simulink model "ofdm_txrx_supermimo"  (Simulink 7.1)
settings: Solver=VariableStepDiscrete  SolverMode=SingleTasking  StartTime=0.0  StopTime=simOnly_simLength  RelTol=1e-3
PostLoadFcn:
  | ofdm_rx_supermimo_init
InitFcn:
  | ofdm_rx_supermimo_init
StartFcn:
  | ofdm_rx_supermimo_init
values from neighbouring files (symbols this model uses):
  Action0_Config = 0* 31*2^18 + 0*2^24 + PHY_AUTORESPONSE_REQ_GOODHDR + PHY_AUTORESPONSE_REQ_GOODPKT + PHY_AUTORESPONSE_REQ_FLAGA    [ofdm_tx_supermimo_init.m]
  Action1_Config = 0* 62*2^18 + PHY_AUTORESPONSE_REQ_GOODHDR + PHY_AUTORESPONSE_REQ_GOODPKT    [ofdm_tx_supermimo_init.m]
  Action2_Config = 0    [ofdm_tx_supermimo_init.m]
  Action3_Config = 0    [ofdm_tx_supermimo_init.m]
  Action4_Config = 0    [ofdm_tx_supermimo_init.m]
  Action5_Config = 0    [ofdm_tx_supermimo_init.m]
  ActionID_AFTransmit = 31    [ofdm_tx_supermimo_init.m]
  ActionID_Disabled = 0    [ofdm_tx_supermimo_init.m]
  ActionID_SetFlagA = 62    [ofdm_tx_supermimo_init.m]
  ActionID_SetFlagB = 61    [ofdm_tx_supermimo_init.m]
  BER_RAM_init_size = 2048    [ofdm_tx_supermimo_init.m]
  CPLength = 16    [ofdm_tx_supermimo_init.m]
  longCorr_coef_i = [-4*real(LTS) 0]    [ofdm_rx_supermimo_init.m]
  longCorr_coef_q = [4*imag(LTS) 0]    [ofdm_rx_supermimo_init.m]
  Match0_Config = 0    [ofdm_tx_supermimo_init.m]
  Match1_Config = 0    [ofdm_tx_supermimo_init.m]
  Match2_Config = 0    [ofdm_tx_supermimo_init.m]
  Match3_Config = 0    [ofdm_tx_supermimo_init.m]
  Match4_Config = 0    [ofdm_tx_supermimo_init.m]
  Match5_Config = 0    [ofdm_tx_supermimo_init.m]
  Match6_Config = 0    [ofdm_tx_supermimo_init.m]
  Match7_Config = 0    [ofdm_tx_supermimo_init.m]
  max_CP_length = 16    [ofdm_tx_supermimo_init.m]
  max_num_baseRateSymbols = 31    [ofdm_tx_supermimo_init.m]
  max_num_subcarriers = 64    [ofdm_tx_supermimo_init.m]
  max_num_trainingSymbols = 15    [ofdm_tx_supermimo_init.m]
  max_numBytes = 16384    [ofdm_tx_supermimo_init.m]
  max_OFDM_symbols = 511    [ofdm_tx_supermimo_init.m]
  modConstellation_qam16 = .75*[1 3 -1 -3]./3    [ofdm_tx_supermimo_init.m]
  modConstellation_qam64 = 0.875*[3 1 5 7 -3 -1 -5 -7]./7    [ofdm_tx_supermimo_init.m]
  modConstellation_qpsk = [1 -1]./sqrt(2)    [ofdm_tx_supermimo_init.m]
  numBaseRateSymbols = ceil(numHeaderBytes / numBytes_BaseRateOFDMSymbol)    [ofdm_tx_supermimo_init.m]
  numSubcarriers = 64    [ofdm_tx_supermimo_init.m]
  numTrainingSymbols = 2    [ofdm_tx_supermimo_init.m]
  pilot_indicies = 7 + ( (64-21) * 2^8) + ( (64-7) * 2^16) + (21 * 2^24)    (= 356068103)    [ofdm_tx_supermimo_init.m]
  pilotValues = hex2dec('A57D') + (2^16 * hex2dec('5A82'))    [ofdm_tx_supermimo_init.m]
  pkt_crossCorr_thresh = 0.7    [ofdm_rx_supermimo_init.m]
  pkt_energy_thresh = 0    [ofdm_rx_supermimo_init.m]
  pktByteNums = numHeaderBytes +  				byteIndex_numPayloadBytes(1)*2^8 +  				byteIndex_numPayloadBytes(2)*2^16 +  				byteIndex_simpleDynModMasks * 2^24    [ofdm_rx_supermimo_init.m]
  PktDet_Delay = 58+32 +      Symbol_Timing_Offset*2^7 +      0*2^16 +      (117* 2^24)    (= 1962935642)    [ofdm_rx_supermimo_init.m]
  pktTiming_count_payloadStart = pktTiming_count_lsStart + pktTiming_ls_num*pktTiming_ls_length    [ofdm_rx_supermimo_init.m]
  preamble = preamble_scale*[shortsyms_10 longsyms_2]    [ofdm_tx_supermimo_init.m]
  preamble_ROM = preamble_I_ROM + preamble_Q_ROM*2^16    [ofdm_tx_supermimo_init.m]
  RAM_init_size = max_numBytes    (= 16384)    [ofdm_tx_supermimo_init.m]
  reg_AF_Tx_Blanking = (320+80) + ( (320+80+79)*2^16)    (= 31392144)    [ofdm_rx_supermimo_init.m]
  reg_AF_Tx_Scaling = 2944    [ofdm_rx_supermimo_init.m]
  reg_Interrupt_PktBuf_Control = reg_InterruptControl +  	1 * 2^16 +  	0 * 2^24    (= 65656)    [ofdm_rx_supermimo_init.m]
  reg_PilotCalcParams = (4 * 2^16) + (minPilotChanMag)    (= 262184.96)    [ofdm_rx_supermimo_init.m]
  regRx_chanEst_minMag = ((0.5)*2^15) + ((0.5 * 2^15)*2^16)    (= 1073758208)    [ofdm_rx_supermimo_init.m]
  regRx_coarseCalcCorrection = round((1.3e-3 - 1.2982e-3)*2^32)    [ofdm_rx_supermimo_init.m]
  regRx_pilotCalcCorrection = round(1.00115 * 2^31)    [ofdm_rx_supermimo_init.m]
  regRx_preCFOoptions = 0 * 2^0 +  	1 * 2^1 +  	0    (= 2)    [ofdm_rx_supermimo_init.m]
  rx_controlBits = 1 * 2  		 + 1 * 4  		 + 0 * 8  		 + 1 * 16 * rx_SISO_Mode  		 + 1 * 32  		 + 1 * 64  		 + 1 * 128  		 + 1 * 256  		 + 1 * 512  		 + 1 * 1024  		 + 1 * 4096  		 + 0 * 16384  		 + 0 * 32768  		 + 1 * 65536  		 + 0 * 131072  		 + 0 * 262144  		 + 1 * 524288  		 + 1 * 1048576 * tx_Alamouti_Mode  		 + 0 * 2097152           + 1 * 33554432  		 + 0 * 4194304  		 + 0 * 8388608  		 + 0 * 16777216  		 + 1 *  ...(+49 chars)    [ofdm_rx_supermimo_init.m]
  Rx_PktDet_LongCorr_Params = LongCorrCounterLoad + 0*2^8 + 0*2^16 + 255*2^24    (= 4278190325)    [ofdm_rx_supermimo_init.m]
  Rx_PktDet_LongCorrThresholds = 8000    [ofdm_rx_supermimo_init.m]
  rx_postEq_scaling = round(rxScaling*2^11) * (1 + 2^16)    [ofdm_rx_supermimo_init.m]
  RxDataScrambling_Seq = [40 198 78 63 82 173 102 245 48 111 172 115 147 230 216 93 72 65 62 2 205 242 122 90 128 83 105 97 73 10 5 252]    [ofdm_tx_supermimo_init.m]
  RxReg_FixedPktLen = 0    [ofdm_rx_supermimo_init.m]
  shortSymbol_time = shortSymbol_time(1:16).'    [ofdm_tx_supermimo_init.m]
  simOnly_numSamples = length(preamble)+( (numSubcarriers+CPLength)*(numTrainingSymbols + numBaseRateSymbols + numFullRateSymbols/2) )    [ofdm_tx_supermimo_init.m]
  train = [train train]    [ofdm_tx_supermimo_init.m]
  tx_controlBits = (antB_preambleShift * 2^4) + (2^1 * tx_Alamouti_Mode) +  	(2^0 * tx_SISO_Mode) + 4*0 + 8*1 + 0*256 + 512*0 + 1024*0 +  	0*hex2dec('1000') + 0*hex2dec('2000') + 0*hex2dec('4000') +  	1*hex2dec('8000') + 1*hex2dec('10000') + hex2dec('20000') +  	16*hex2dec('100000')    [ofdm_tx_supermimo_init.m]
  tx_delays = (50*2^0) + (0*2^8) + (30*2^12)    (= 122930)    [ofdm_tx_supermimo_init.m]
  tx_scaling = (3072) + (12288 * 2^16)    (= 805309440)    [ofdm_tx_supermimo_init.m]
  TxDataScrambling_Seq = [40 198 78 63 82 173 102 245 48 111 172 115 147 230 216 93 72 65 62 2 205 242 122 90 128 83 105 97 73 10 5 252]    [ofdm_tx_supermimo_init.m]
  txfilt_firpm = 0.95*txfilt_firpm./(max(txfilt_firpm))    [ofdm_tx_supermimo_init.m]
  txfilt_intfilt = [0 intfilt(4,4,.8)]    [ofdm_tx_supermimo_init.m]
  txReg_symbolCounts = (2^8 *numBaseRateSymbols ) + numTrainingSymbols    [ofdm_tx_supermimo_init.m]
  TxRx_FFTScaling = (3 * 2^0) +  	(2 * 2^2) +  	(1 * 2^4) +  	(1 * 2^6) +  	(1 * 2^8) +  	(0 * 2^10)    (= 347)    [ofdm_tx_supermimo_init.m]
undefined symbols (not defined in the file or in its folder): AntA, AntB, BaseRate, Down, gcbh, numBuffs, numBytes, Up, xlBlockLoadCallback, xlGetSimulinkPeriod, xlipgetpartsetting
libraries: xbsIndex_r4 x6035, simulink x4, dspsigops x4, commchan3 x2, dspsrcs4 x2, commrflib2 x2
(part 21 of 61)

== system ofdm_txrx_supermimo/OFDM Rx MIMO/FFT & Chan Est/FFT/FreqSelective "Fading" ==
  I : Inport
  Q : Inport  Port=2
  Ind : Inport  Port=3
  "FreqSelective \"Fading\"" : Subsystem
  Simulation Multiplexer : xbsIndex_r4/Simulation Multiplexer  sim_sel=1  hw_sel=2  block_type=simmux
      settings: infoedit="Distinguish input subsystems as \"simulation only\" and \"generation only\".  The input specified For Simulation will be used during Simulink simulation.  The input specified For Generation will be used during code generation.  This block will typically be used on the output of a HW Co-Simulation, Black Box, or ModelSim subsystem.<P><P>Hardware Notes: This block costs nothing."  block_version=10.1.3
  Simulation Multiplexer1 : xbsIndex_r4/Simulation Multiplexer  sim_sel=1  hw_sel=2  block_type=simmux
      settings: infoedit="Distinguish input subsystems as \"simulation only\" and \"generation only\".  The input specified For Simulation will be used during Simulink simulation.  The input specified For Generation will be used during code generation.  This block will typically be used on the output of a HW Co-Simulation, Black Box, or ModelSim subsystem.<P><P>Hardware Notes: This block costs nothing."  block_version=10.1.3
  " I" : Outport  OutDataType=sfix(16)  OutScaling=2^0
  " Q" : Outport  Port=2  OutDataType=sfix(16)  OutScaling=2^0
  wires:
  "FreqSelective \"Fading\""/1(" I") -> Simulation Multiplexer/1
  "FreqSelective \"Fading\""/2(" Q") -> Simulation Multiplexer1/1
  I/1 -> "FreqSelective \"Fading\""/1(I), Simulation Multiplexer/2
  Q/1 -> "FreqSelective \"Fading\""/2(Q), Simulation Multiplexer1/2
  Ind/1 -> "FreqSelective \"Fading\""/3(Ind)
  Simulation Multiplexer/1 -> " I"/1
  Simulation Multiplexer1/1 -> " Q"/1

== system ofdm_txrx_supermimo/OFDM Rx MIMO/FFT & Chan Est/Input Buffers/Antenna A RAM ==
  I : Inport  OutDataType=sfix(16)  OutScaling=2^0
  Q : Inport  Port=2  OutDataType=sfix(16)  OutScaling=2^0
  We : Inport  Port=3  OutDataType=sfix(16)  OutScaling=2^0
  Wr Addr : Inport  Port=4  OutDataType=sfix(16)  OutScaling=2^0
  Rd Addr : Inport  Port=5  OutDataType=sfix(16)  OutScaling=2^0
  Constant8 : xbsIndex_r4/Constant  arith_type=Boolean  const=0  n_bits=1  bin_pt=0  explicit_period=off  period=1  equ=P=C  opselect=C  inp2=PCIN>>17  opr=+  inp1=P  carry=CIN  dbl_ovrd=off  block_type=constant
      settings: block_version=VER_STRING_GOES_HERE
  Dual Port RAM : xbsIndex_r4/Dual Port RAM  depth=2*numSubcarriers  initVector=[0.01:0.01:0.80 ones(1,48)]  distributed_mem=Block RAM  init_a=0  init_b=0  rst_a=off  rst_b=off  en_a=off  en_b=off  latency=1  write_mode_A=Read After Write  write_mode_B=Read After Write  dbl_ovrd=off  optimize=Area  use_rpm=off  block_type=dpram
      settings: xl_area=[0 0 1 0 0 0 0]  block_version=VER_STRING_GOES_HERE
  Dual Port RAM1 : xbsIndex_r4/Dual Port RAM  depth=2*numSubcarriers  initVector=[0.80:-0.01:0.01 ones(1,48)]  distributed_mem=Block RAM  init_a=0  init_b=0  rst_a=off  rst_b=off  en_a=off  en_b=off  latency=1  write_mode_A=Read After Write  write_mode_B=Read After Write  dbl_ovrd=off  optimize=Area  use_rpm=off  block_type=dpram
      settings: xl_area=[0 0 1 0 0 0 0]  block_version=VER_STRING_GOES_HERE
  " I" : Outport  OutDataType=sfix(16)  OutScaling=2^0
  " Q" : Outport  Port=2  OutDataType=sfix(16)  OutScaling=2^0
  wires:
  Wr Addr/1 -> Dual Port RAM1/1, Dual Port RAM/1
  Constant8/1 -> Dual Port RAM/6, Dual Port RAM1/6
  Rd Addr/1 -> Dual Port RAM1/4, Dual Port RAM/4
  Dual Port RAM1/2 -> " Q"/1
  Dual Port RAM/2 -> " I"/1
  I/1 -> Dual Port RAM/2, Dual Port RAM/5, Dual Port RAM1/5
  Q/1 -> Dual Port RAM1/2
  We/1 -> Dual Port RAM1/3, Dual Port RAM/3

== system ofdm_txrx_supermimo/OFDM Rx MIMO/FFT & Chan Est/Input Buffers/Antenna B RAM ==
  I : Inport  OutDataType=sfix(16)  OutScaling=2^0
  Q : Inport  Port=2  OutDataType=sfix(16)  OutScaling=2^0
  We : Inport  Port=3  OutDataType=sfix(16)  OutScaling=2^0
  Wr Addr : Inport  Port=4  OutDataType=sfix(16)  OutScaling=2^0
  Rd Addr : Inport  Port=5  OutDataType=sfix(16)  OutScaling=2^0
  Constant8 : xbsIndex_r4/Constant  arith_type=Boolean  const=0  n_bits=1  bin_pt=0  explicit_period=off  period=1  equ=P=C  opselect=C  inp2=PCIN>>17  opr=+  inp1=P  carry=CIN  dbl_ovrd=off  block_type=constant
      settings: block_version=VER_STRING_GOES_HERE
  Dual Port RAM : xbsIndex_r4/Dual Port RAM  depth=2*numSubcarriers  initVector=[0.01:0.01:0.80 ones(1,48)]  distributed_mem=Block RAM  init_a=0  init_b=0  rst_a=off  rst_b=off  en_a=off  en_b=off  latency=1  write_mode_A=Read After Write  write_mode_B=Read After Write  dbl_ovrd=off  optimize=Area  use_rpm=off  block_type=dpram
      settings: xl_area=[0 0 1 0 0 0 0]  block_version=VER_STRING_GOES_HERE
  Dual Port RAM1 : xbsIndex_r4/Dual Port RAM  depth=2*numSubcarriers  initVector=[0.80:-0.01:0.01 ones(1,48)]  distributed_mem=Block RAM  init_a=0  init_b=0  rst_a=off  rst_b=off  en_a=off  en_b=off  latency=1  write_mode_A=Read After Write  write_mode_B=Read After Write  dbl_ovrd=off  optimize=Area  use_rpm=off  block_type=dpram
      settings: xl_area=[0 0 1 0 0 0 0]  block_version=VER_STRING_GOES_HERE
  " I" : Outport  OutDataType=sfix(16)  OutScaling=2^0
  " Q" : Outport  Port=2  OutDataType=sfix(16)  OutScaling=2^0
  wires:
  We/1 -> Dual Port RAM/3, Dual Port RAM1/3
  Q/1 -> Dual Port RAM1/2
  I/1 -> Dual Port RAM/2, Dual Port RAM/5, Dual Port RAM1/5
  Dual Port RAM/2 -> " I"/1
  Dual Port RAM1/2 -> " Q"/1
  Rd Addr/1 -> Dual Port RAM/4, Dual Port RAM1/4
  Constant8/1 -> Dual Port RAM1/6, Dual Port RAM/6
  Wr Addr/1 -> Dual Port RAM/1, Dual Port RAM1/1

== system ofdm_txrx_supermimo/OFDM Rx MIMO/FFT & Chan Est/Input Buffers/We Control ==
  note: In Alamouti mode, alternate symbols
      | are written to alternate memory buffers.
      | In MIMO mode, symbols are written to buffers
      | simultaneously (from each antenna).
  We : Inport
  WrSlot : Inport  Port=2
  Down Sample : xbsIndex_r4/Down Sample  sample_ratio=2  sample_phase="Last Value of Frame  (most efficient)"  en=off  latency=1  dbl_ovrd=off  block_type=dsamp
      settings: block_version=VER_STRING_GOES_HERE
  Inverter1 : xbsIndex_r4/Inverter  en=off  latency=0  dbl_ovrd=off  block_type=inv
      settings: block_version=VER_STRING_GOES_HERE
  Inverter2 : xbsIndex_r4/Inverter  en=off  latency=0  dbl_ovrd=off  block_type=inv
      settings: block_version=VER_STRING_GOES_HERE
  Inverter3 : xbsIndex_r4/Inverter  en=off  latency=0  dbl_ovrd=off  block_type=inv
      settings: block_version=VER_STRING_GOES_HERE
  Logical1 : xbsIndex_r4/Logical  logical_function=AND  inputs=3  en=off  latency=0  precision=Full  arith_type=Unsigned  n_bits=16  bin_pt=0  align_bp=on  dbl_ovrd=off  block_type=logical
      settings: block_version=8.2.02
  Logical3 : xbsIndex_r4/Logical  logical_function=AND  inputs=3  en=off  latency=0  precision=Full  arith_type=Unsigned  n_bits=16  bin_pt=0  align_bp=on  dbl_ovrd=off  block_type=logical
      settings: block_version=8.2.02
  Logical4 : xbsIndex_r4/Logical  logical_function=OR  inputs=2  en=off  latency=0  precision=Full  arith_type=Unsigned  n_bits=16  bin_pt=0  align_bp=on  dbl_ovrd=off  block_type=logical
      settings: block_version=8.2.02
  Logical5 : xbsIndex_r4/Logical  logical_function=AND  inputs=2  en=off  latency=0  precision=Full  arith_type=Unsigned  n_bits=16  bin_pt=0  align_bp=on  dbl_ovrd=off  block_type=logical
      settings: block_version=8.2.02
  Logical6 : xbsIndex_r4/Logical  logical_function=AND  inputs=2  en=off  latency=0  precision=Full  arith_type=Unsigned  n_bits=16  bin_pt=0  align_bp=on  dbl_ovrd=off  block_type=logical
      settings: block_version=8.2.02
  Logical7 : xbsIndex_r4/Logical  logical_function=OR  inputs=2  en=off  latency=0  precision=Full  arith_type=Unsigned  n_bits=16  bin_pt=0  align_bp=on  dbl_ovrd=off  block_type=logical
      settings: block_version=8.2.02
  A_We : Outport
  B_We : Outport  Port=2
  wires:
  We/1 -> Logical3/1, Logical1/1, Logical6/1, Logical5/1
  WrSlot/1 -> Inverter1/1, Logical3/2
  Inverter1/1 -> Logical1/2
  [regRx_AlamoutiMode] -> Down Sample/1
  Inverter2/1 -> Logical5/2
  Inverter3/1 -> Logical6/2
  Logical5/1 -> Logical4/1
  Logical3/1 -> Logical4/2
  Logical4/1 -> B_We/1
  Logical6/1 -> Logical7/1
  Logical1/1 -> Logical7/2
  Logical7/1 -> A_We/1
  Down Sample/1 -> Logical1/3, Inverter3/1, Logical3/3, Inverter2/1

== system ofdm_txrx_supermimo/OFDM Rx MIMO/FFT & Chan Est/Output Buffers/AntA Buffer ==
  I : Inport  OutDataType=sfix(16)  OutScaling=2^0
  Q : Inport  Port=2  OutDataType=sfix(16)  OutScaling=2^0
  We : Inport  Port=3  OutDataType=sfix(16)  OutScaling=2^0
  Wr Addr : Inport  Port=4  OutDataType=sfix(16)  OutScaling=2^0
  Rd Addr : Inport  Port=5  OutDataType=sfix(16)  OutScaling=2^0
  Constant : xbsIndex_r4/Constant  arith_type=Signed (2's comp)  const=0  n_bits=16  bin_pt=15  explicit_period=on  period=2  equ=P=C  opselect=C  inp2=PCIN>>17  opr=+  inp1=P  carry=CIN  dbl_ovrd=off  block_type=constant
      settings: block_version=10.1.2
  Constant8 : xbsIndex_r4/Constant  arith_type=Boolean  const=0  n_bits=1  bin_pt=0  explicit_period=off  period=1  equ=P=C  opselect=C  inp2=PCIN>>17  opr=+  inp1=P  carry=CIN  dbl_ovrd=off  block_type=constant
      settings: block_version=VER_STRING_GOES_HERE
  Dual Port RAM : xbsIndex_r4/Dual Port RAM  depth=numSubcarriers  initVector=[0.01:0.01:0.80 ones(1,48)]  distributed_mem=Block RAM  init_a=0  init_b=0  rst_a=off  rst_b=off  en_a=off  en_b=off  latency=1  write_mode_A=Read After Write  write_mode_B=Read After Write  dbl_ovrd=off  optimize=Area  use_rpm=off  block_type=dpram
      settings: xl_area=[0 0 1 0 0 0 0]  block_version=VER_STRING_GOES_HERE
  Dual Port RAM1 : xbsIndex_r4/Dual Port RAM  depth=numSubcarriers  initVector=[0.80:-0.01:0.01 ones(1,48)]  distributed_mem=Block RAM  init_a=0  init_b=0  rst_a=off  rst_b=off  en_a=off  en_b=off  latency=1  write_mode_A=Read After Write  write_mode_B=Read After Write  dbl_ovrd=off  optimize=Area  use_rpm=off  block_type=dpram
      settings: xl_area=[0 0 1 0 0 0 0]  block_version=VER_STRING_GOES_HERE
  " I" : Outport  OutDataType=sfix(16)  OutScaling=2^0
  " Q" : Outport  Port=2  OutDataType=sfix(16)  OutScaling=2^0
  wires:
  We/1 -> Dual Port RAM/3, Dual Port RAM1/3
  Q/1 -> Dual Port RAM1/2
  I/1 -> Dual Port RAM/2
  Dual Port RAM/2 -> " I"/1
  Dual Port RAM1/2 -> " Q"/1
  Rd Addr/1 -> Dual Port RAM/4, Dual Port RAM1/4
  Constant8/1 -> Dual Port RAM1/6, Dual Port RAM/6
  Wr Addr/1 -> Dual Port RAM/1, Dual Port RAM1/1
  Constant/1 -> Dual Port RAM/5, Dual Port RAM1/5

== system ofdm_txrx_supermimo/OFDM Rx MIMO/FFT & Chan Est/Output Buffers/AntB Buffer ==
  I : Inport  OutDataType=sfix(16)  OutScaling=2^0
  Q : Inport  Port=2  OutDataType=sfix(16)  OutScaling=2^0
  We : Inport  Port=3  OutDataType=sfix(16)  OutScaling=2^0
  Wr Addr : Inport  Port=4  OutDataType=sfix(16)  OutScaling=2^0
  Rd Addr : Inport  Port=5  OutDataType=sfix(16)  OutScaling=2^0
  Constant : xbsIndex_r4/Constant  arith_type=Signed (2's comp)  const=0  n_bits=16  bin_pt=15  explicit_period=on  period=2  equ=P=C  opselect=C  inp2=PCIN>>17  opr=+  inp1=P  carry=CIN  dbl_ovrd=off  block_type=constant
      settings: block_version=10.1.2
  Constant8 : xbsIndex_r4/Constant  arith_type=Boolean  const=0  n_bits=1  bin_pt=0  explicit_period=off  period=1  equ=P=C  opselect=C  inp2=PCIN>>17  opr=+  inp1=P  carry=CIN  dbl_ovrd=off  block_type=constant
      settings: block_version=VER_STRING_GOES_HERE
  Dual Port RAM : xbsIndex_r4/Dual Port RAM  depth=numSubcarriers  initVector=[0.01:0.01:0.80 ones(1,48)]  distributed_mem=Block RAM  init_a=0  init_b=0  rst_a=off  rst_b=off  en_a=off  en_b=off  latency=1  write_mode_A=Read After Write  write_mode_B=Read After Write  dbl_ovrd=off  optimize=Area  use_rpm=off  block_type=dpram
      settings: xl_area=[0 0 1 0 0 0 0]  block_version=VER_STRING_GOES_HERE
  Dual Port RAM1 : xbsIndex_r4/Dual Port RAM  depth=numSubcarriers  initVector=[0.80:-0.01:0.01 ones(1,48)]  distributed_mem=Block RAM  init_a=0  init_b=0  rst_a=off  rst_b=off  en_a=off  en_b=off  latency=1  write_mode_A=Read After Write  write_mode_B=Read After Write  dbl_ovrd=off  optimize=Area  use_rpm=off  block_type=dpram
      settings: xl_area=[0 0 1 0 0 0 0]  block_version=VER_STRING_GOES_HERE
  " I" : Outport  OutDataType=sfix(16)  OutScaling=2^0
  " Q" : Outport  Port=2  OutDataType=sfix(16)  OutScaling=2^0
  wires:
  Wr Addr/1 -> Dual Port RAM1/1, Dual Port RAM/1
  Constant8/1 -> Dual Port RAM/6, Dual Port RAM1/6
  Rd Addr/1 -> Dual Port RAM1/4, Dual Port RAM/4
  Dual Port RAM1/2 -> " Q"/1
  Dual Port RAM/2 -> " I"/1
  I/1 -> Dual Port RAM/2
  Q/1 -> Dual Port RAM1/2
  We/1 -> Dual Port RAM1/3, Dual Port RAM/3
  Constant/1 -> Dual Port RAM/5, Dual Port RAM1/5

== system ofdm_txrx_supermimo/OFDM Rx MIMO/FFT & Chan Est/Symbol Counter/S-R Latch ==
  S : Inport  OutDataType=sfix(16)  OutScaling=2^0
  R : Inport  Port=2  OutDataType=sfix(16)  OutScaling=2^0
  Constant2 : xbsIndex_r4/Constant  arith_type=Boolean  const=1  n_bits=1  bin_pt=0  explicit_period=off  period=1  equ=P=C  opselect=C  inp2=PCIN>>17  opr=+  inp1=P  carry=CIN  dbl_ovrd=off  block_type=constant
      settings: block_version=VER_STRING_GOES_HERE
  Register : xbsIndex_r4/Register  init=0  rst=on  en=on  dbl_ovrd=off  block_type=register
      settings: xl_area=[0, 0, 0, 0, 0, 0, 0]  block_version=VER_STRING_GOES_HERE
  D : Outport  OutDataType=sfix(16)  OutScaling=2^0
  wires:
  Register/1 -> D/1
  S/1 -> Register/3
  R/1 -> Register/2
  Constant2/1 -> Register/1

== system ofdm_txrx_supermimo/OFDM Rx MIMO/Long Corr HP/C1/C1 ==
  xn : Inport  OutDataType=sfix(16)  OutScaling=2^0
  DAddr : Inport  Port=2  OutDataType=sfix(16)  OutScaling=2^0
  CAddr : Inport  Port=3  OutDataType=sfix(16)  OutScaling=2^0
  LD : Inport  Port=4  OutDataType=sfix(16)  OutScaling=2^0
  Down Sample2 : xbsIndex_r4/Down Sample  sample_ratio=length(coef2)  sample_phase=First Value of Frame  en=off  latency=0  dbl_ovrd=off  block_type=dsamp
      settings: xl_area=[5 5 0 4 0 0 0]  block_version=VER_STRING_GOES_HERE
  MAC : Subsystem
  R : xbsIndex_r4/Addressable Shift Register  infer_latency=off  depth=length(coef2)  initVector=[0]  en=off  dbl_ovrd=off  use_behavioral_HDL=off  use_rpm=off  block_type=addrsr
      settings: infoedit="Delay of  configurable length.  Any element  in the delay     line   can    be   addressed   and   driven    onto   the   output     port.<P><P>Hardware  notes: Implemented  using SRL16s.     If Virtex-4, Virtex-II or Spartan-3 devices are used, multiple SRLC16s are cascaded together."  xl_area=[1 0 0 2 0 0 0]  block_version=VER_STRING_GOES_HERE
  ROM1 : xbsIndex_r4/ROM  depth=length(coef2)  initVector=coef2  distributed_mem=Distributed memory  rst=off  init_reg=0  en=off  latency=1  arith_type="Signed  (2's comp)"  n_bits=longCorr_coef_nbits  bin_pt=longCorr_coef_bp  dbl_ovrd=off  optimize=Area  use_rpm=off  block_type=sprom
      settings: xl_area=[1 1 0 1 0 0 0]  block_version=VER_STRING_GOES_HERE
  yn : Outport  OutDataType=sfix(16)  OutScaling=2^0
  xnz : Outport  Port=2  OutDataType=sfix(16)  OutScaling=2^0
  wires:
  xn/1 -> R/1
  DAddr/1 -> R/2
  CAddr/1 -> ROM1/1
  R/1 -> xnz/1, MAC/1(a)
  MAC/1(yn) -> Down Sample2/1
  Down Sample2/1 -> yn/1
  ROM1/1 -> MAC/2(coeff)
  LD/1 -> MAC/3(ld)

== system ofdm_txrx_supermimo/OFDM Rx MIMO/Long Corr HP/C1/C10 ==
  xn : Inport  OutDataType=sfix(16)  OutScaling=2^0
  DAddr : Inport  Port=2  OutDataType=sfix(16)  OutScaling=2^0
  CAddr : Inport  Port=3  OutDataType=sfix(16)  OutScaling=2^0
  LD : Inport  Port=4  OutDataType=sfix(16)  OutScaling=2^0
  Down Sample1 : xbsIndex_r4/Down Sample  sample_ratio=length(coef2)  sample_phase=First Value of Frame  en=off  latency=0  dbl_ovrd=off  block_type=dsamp
      settings: xl_area=[3 3 0 2 0 0 0]  block_version=VER_STRING_GOES_HERE
  Down Sample2 : xbsIndex_r4/Down Sample  sample_ratio=length(coef2)  sample_phase=First Value of Frame  en=off  latency=0  dbl_ovrd=off  block_type=dsamp
      settings: xl_area=[5 5 0 4 0 0 0]  block_version=VER_STRING_GOES_HERE
  MAC : Subsystem
  R : xbsIndex_r4/Addressable Shift Register  infer_latency=off  depth=length(coef2)  initVector=[0]  en=off  dbl_ovrd=off  use_behavioral_HDL=off  use_rpm=off  block_type=addrsr
      settings: infoedit="Delay of  configurable length.  Any element  in the delay     line   can    be   addressed   and   driven    onto   the   output     port.<P><P>Hardware  notes: Implemented  using SRL16s.     If Virtex-4, Virtex-II or Spartan-3 devices are used, multiple SRLC16s are cascaded together."  xl_area=[1 0 0 2 0 0 0]  block_version=VER_STRING_GOES_HERE
  ROM1 : xbsIndex_r4/ROM  depth=length(coef2)  initVector=coef2  distributed_mem=Distributed memory  rst=off  init_reg=0  en=off  latency=1  arith_type="Signed  (2's comp)"  n_bits=longCorr_coef_nbits  bin_pt=longCorr_coef_bp  dbl_ovrd=off  optimize=Area  use_rpm=off  block_type=sprom
      settings: xl_area=[1 1 0 1 0 0 0]  block_version=VER_STRING_GOES_HERE
  yn : Outport  OutDataType=sfix(16)  OutScaling=2^0
  xnz : Outport  Port=2  OutDataType=sfix(16)  OutScaling=2^0
  wires:
  Down Sample1/1 -> R/1
  xn/1 -> Down Sample1/1
  DAddr/1 -> R/2
  CAddr/1 -> ROM1/1
  R/1 -> xnz/1, MAC/1(a)
  MAC/1(yn) -> Down Sample2/1
  Down Sample2/1 -> yn/1
  ROM1/1 -> MAC/2(coeff)
  LD/1 -> MAC/3(ld)

== system ofdm_txrx_supermimo/OFDM Rx MIMO/Long Corr HP/C1/C11 ==
  xn : Inport  OutDataType=sfix(16)  OutScaling=2^0
  DAddr : Inport  Port=2  OutDataType=sfix(16)  OutScaling=2^0
  CAddr : Inport  Port=3  OutDataType=sfix(16)  OutScaling=2^0
  LD : Inport  Port=4  OutDataType=sfix(16)  OutScaling=2^0
  Down Sample1 : xbsIndex_r4/Down Sample  sample_ratio=length(coef2)  sample_phase=First Value of Frame  en=off  latency=0  dbl_ovrd=off  block_type=dsamp
      settings: xl_area=[3 3 0 2 0 0 0]  block_version=VER_STRING_GOES_HERE
  Down Sample2 : xbsIndex_r4/Down Sample  sample_ratio=length(coef2)  sample_phase=First Value of Frame  en=off  latency=0  dbl_ovrd=off  block_type=dsamp
      settings: xl_area=[5 5 0 4 0 0 0]  block_version=VER_STRING_GOES_HERE
  MAC : Subsystem
  R : xbsIndex_r4/Addressable Shift Register  infer_latency=off  depth=length(coef2)  initVector=[0]  en=off  dbl_ovrd=off  use_behavioral_HDL=off  use_rpm=off  block_type=addrsr
      settings: infoedit="Delay of  configurable length.  Any element  in the delay     line   can    be   addressed   and   driven    onto   the   output     port.<P><P>Hardware  notes: Implemented  using SRL16s.     If Virtex-4, Virtex-II or Spartan-3 devices are used, multiple SRLC16s are cascaded together."  xl_area=[1 0 0 2 0 0 0]  block_version=VER_STRING_GOES_HERE
  ROM1 : xbsIndex_r4/ROM  depth=length(coef2)  initVector=coef2  distributed_mem=Distributed memory  rst=off  init_reg=0  en=off  latency=1  arith_type="Signed  (2's comp)"  n_bits=longCorr_coef_nbits  bin_pt=longCorr_coef_bp  dbl_ovrd=off  optimize=Area  use_rpm=off  block_type=sprom
      settings: xl_area=[1 1 0 1 0 0 0]  block_version=VER_STRING_GOES_HERE
  yn : Outport  OutDataType=sfix(16)  OutScaling=2^0
  xnz : Outport  Port=2  OutDataType=sfix(16)  OutScaling=2^0
  wires:
  Down Sample1/1 -> R/1
  xn/1 -> Down Sample1/1
  DAddr/1 -> R/2
  CAddr/1 -> ROM1/1
  R/1 -> xnz/1, MAC/1(a)
  MAC/1(yn) -> Down Sample2/1
  Down Sample2/1 -> yn/1
  ROM1/1 -> MAC/2(coeff)
  LD/1 -> MAC/3(ld)

== system ofdm_txrx_supermimo/OFDM Rx MIMO/Long Corr HP/C1/C12 ==
  xn : Inport  OutDataType=sfix(16)  OutScaling=2^0
  DAddr : Inport  Port=2  OutDataType=sfix(16)  OutScaling=2^0
  CAddr : Inport  Port=3  OutDataType=sfix(16)  OutScaling=2^0
  LD : Inport  Port=4  OutDataType=sfix(16)  OutScaling=2^0
  Down Sample1 : xbsIndex_r4/Down Sample  sample_ratio=length(coef2)  sample_phase=First Value of Frame  en=off  latency=0  dbl_ovrd=off  block_type=dsamp
      settings: xl_area=[3 3 0 2 0 0 0]  block_version=VER_STRING_GOES_HERE
  Down Sample2 : xbsIndex_r4/Down Sample  sample_ratio=length(coef2)  sample_phase=First Value of Frame  en=off  latency=0  dbl_ovrd=off  block_type=dsamp
      settings: xl_area=[5 5 0 4 0 0 0]  block_version=VER_STRING_GOES_HERE
  MAC : Subsystem
  R : xbsIndex_r4/Addressable Shift Register  infer_latency=off  depth=length(coef2)  initVector=[0]  en=off  dbl_ovrd=off  use_behavioral_HDL=off  use_rpm=off  block_type=addrsr
      settings: infoedit="Delay of  configurable length.  Any element  in the delay     line   can    be   addressed   and   driven    onto   the   output     port.<P><P>Hardware  notes: Implemented  using SRL16s.     If Virtex-4, Virtex-II or Spartan-3 devices are used, multiple SRLC16s are cascaded together."  xl_area=[1 0 0 2 0 0 0]  block_version=VER_STRING_GOES_HERE
  ROM1 : xbsIndex_r4/ROM  depth=length(coef2)  initVector=coef2  distributed_mem=Distributed memory  rst=off  init_reg=0  en=off  latency=1  arith_type="Signed  (2's comp)"  n_bits=longCorr_coef_nbits  bin_pt=longCorr_coef_bp  dbl_ovrd=off  optimize=Area  use_rpm=off  block_type=sprom
      settings: xl_area=[1 1 0 1 0 0 0]  block_version=VER_STRING_GOES_HERE
  yn : Outport  OutDataType=sfix(16)  OutScaling=2^0
  xnz : Outport  Port=2  OutDataType=sfix(16)  OutScaling=2^0
  wires:
  Down Sample1/1 -> R/1
  xn/1 -> Down Sample1/1
  DAddr/1 -> R/2
  CAddr/1 -> ROM1/1
  R/1 -> xnz/1, MAC/1(a)
  MAC/1(yn) -> Down Sample2/1
  Down Sample2/1 -> yn/1
  ROM1/1 -> MAC/2(coeff)
  LD/1 -> MAC/3(ld)

== system ofdm_txrx_supermimo/OFDM Rx MIMO/Long Corr HP/C1/C13 ==
  xn : Inport  OutDataType=sfix(16)  OutScaling=2^0
  DAddr : Inport  Port=2  OutDataType=sfix(16)  OutScaling=2^0
  CAddr : Inport  Port=3  OutDataType=sfix(16)  OutScaling=2^0
  LD : Inport  Port=4  OutDataType=sfix(16)  OutScaling=2^0
  Down Sample1 : xbsIndex_r4/Down Sample  sample_ratio=length(coef2)  sample_phase=First Value of Frame  en=off  latency=0  dbl_ovrd=off  block_type=dsamp
      settings: xl_area=[3 3 0 2 0 0 0]  block_version=VER_STRING_GOES_HERE
  Down Sample2 : xbsIndex_r4/Down Sample  sample_ratio=length(coef2)  sample_phase=First Value of Frame  en=off  latency=0  dbl_ovrd=off  block_type=dsamp
      settings: xl_area=[5 5 0 4 0 0 0]  block_version=VER_STRING_GOES_HERE
  MAC : Subsystem
  R : xbsIndex_r4/Addressable Shift Register  infer_latency=off  depth=length(coef2)  initVector=[0]  en=off  dbl_ovrd=off  use_behavioral_HDL=off  use_rpm=off  block_type=addrsr
      settings: infoedit="Delay of  configurable length.  Any element  in the delay     line   can    be   addressed   and   driven    onto   the   output     port.<P><P>Hardware  notes: Implemented  using SRL16s.     If Virtex-4, Virtex-II or Spartan-3 devices are used, multiple SRLC16s are cascaded together."  xl_area=[1 0 0 2 0 0 0]  block_version=VER_STRING_GOES_HERE
  ROM1 : xbsIndex_r4/ROM  depth=length(coef2)  initVector=coef2  distributed_mem=Distributed memory  rst=off  init_reg=0  en=off  latency=1  arith_type="Signed  (2's comp)"  n_bits=longCorr_coef_nbits  bin_pt=longCorr_coef_bp  dbl_ovrd=off  optimize=Area  use_rpm=off  block_type=sprom
      settings: xl_area=[1 1 0 1 0 0 0]  block_version=VER_STRING_GOES_HERE
  yn : Outport  OutDataType=sfix(16)  OutScaling=2^0
  xnz : Outport  Port=2  OutDataType=sfix(16)  OutScaling=2^0
  wires:
  Down Sample1/1 -> R/1
  xn/1 -> Down Sample1/1
  DAddr/1 -> R/2
  CAddr/1 -> ROM1/1
  R/1 -> xnz/1, MAC/1(a)
  MAC/1(yn) -> Down Sample2/1
  Down Sample2/1 -> yn/1
  ROM1/1 -> MAC/2(coeff)
  LD/1 -> MAC/3(ld)

== system ofdm_txrx_supermimo/OFDM Rx MIMO/Long Corr HP/C1/C14 ==
  xn : Inport  OutDataType=sfix(16)  OutScaling=2^0
  DAddr : Inport  Port=2  OutDataType=sfix(16)  OutScaling=2^0
  CAddr : Inport  Port=3  OutDataType=sfix(16)  OutScaling=2^0
  LD : Inport  Port=4  OutDataType=sfix(16)  OutScaling=2^0
  Down Sample1 : xbsIndex_r4/Down Sample  sample_ratio=length(coef2)  sample_phase=First Value of Frame  en=off  latency=0  dbl_ovrd=off  block_type=dsamp
      settings: xl_area=[3 3 0 2 0 0 0]  block_version=VER_STRING_GOES_HERE
  Down Sample2 : xbsIndex_r4/Down Sample  sample_ratio=length(coef2)  sample_phase=First Value of Frame  en=off  latency=0  dbl_ovrd=off  block_type=dsamp
      settings: xl_area=[5 5 0 4 0 0 0]  block_version=VER_STRING_GOES_HERE
  MAC : Subsystem
  R : xbsIndex_r4/Addressable Shift Register  infer_latency=off  depth=length(coef2)  initVector=[0]  en=off  dbl_ovrd=off  use_behavioral_HDL=off  use_rpm=off  block_type=addrsr
      settings: infoedit="Delay of  configurable length.  Any element  in the delay     line   can    be   addressed   and   driven    onto   the   output     port.<P><P>Hardware  notes: Implemented  using SRL16s.     If Virtex-4, Virtex-II or Spartan-3 devices are used, multiple SRLC16s are cascaded together."  xl_area=[1 0 0 2 0 0 0]  block_version=VER_STRING_GOES_HERE
  ROM1 : xbsIndex_r4/ROM  depth=length(coef2)  initVector=coef2  distributed_mem=Distributed memory  rst=off  init_reg=0  en=off  latency=1  arith_type="Signed  (2's comp)"  n_bits=longCorr_coef_nbits  bin_pt=longCorr_coef_bp  dbl_ovrd=off  optimize=Area  use_rpm=off  block_type=sprom
      settings: xl_area=[1 1 0 1 0 0 0]  block_version=VER_STRING_GOES_HERE
  yn : Outport  OutDataType=sfix(16)  OutScaling=2^0
  xnz : Outport  Port=2  OutDataType=sfix(16)  OutScaling=2^0
  wires:
  LD/1 -> MAC/3(ld)
  ROM1/1 -> MAC/2(coeff)
  Down Sample2/1 -> yn/1
  MAC/1(yn) -> Down Sample2/1
  R/1 -> xnz/1, MAC/1(a)
  CAddr/1 -> ROM1/1
  DAddr/1 -> R/2
  xn/1 -> Down Sample1/1
  Down Sample1/1 -> R/1

== system ofdm_txrx_supermimo/OFDM Rx MIMO/Long Corr HP/C1/C15 ==
  xn : Inport  OutDataType=sfix(16)  OutScaling=2^0
  DAddr : Inport  Port=2  OutDataType=sfix(16)  OutScaling=2^0
  CAddr : Inport  Port=3  OutDataType=sfix(16)  OutScaling=2^0
  LD : Inport  Port=4  OutDataType=sfix(16)  OutScaling=2^0
  Down Sample1 : xbsIndex_r4/Down Sample  sample_ratio=length(coef2)  sample_phase=First Value of Frame  en=off  latency=0  dbl_ovrd=off  block_type=dsamp
      settings: xl_area=[3 3 0 2 0 0 0]  block_version=VER_STRING_GOES_HERE
  Down Sample2 : xbsIndex_r4/Down Sample  sample_ratio=length(coef2)  sample_phase=First Value of Frame  en=off  latency=0  dbl_ovrd=off  block_type=dsamp
      settings: xl_area=[5 5 0 4 0 0 0]  block_version=VER_STRING_GOES_HERE
  MAC : Subsystem
  R : xbsIndex_r4/Addressable Shift Register  infer_latency=off  depth=length(coef2)  initVector=[0]  en=off  dbl_ovrd=off  use_behavioral_HDL=off  use_rpm=off  block_type=addrsr
      settings: infoedit="Delay of  configurable length.  Any element  in the delay     line   can    be   addressed   and   driven    onto   the   output     port.<P><P>Hardware  notes: Implemented  using SRL16s.     If Virtex-4, Virtex-II or Spartan-3 devices are used, multiple SRLC16s are cascaded together."  xl_area=[1 0 0 2 0 0 0]  block_version=VER_STRING_GOES_HERE
  ROM1 : xbsIndex_r4/ROM  depth=length(coef2)  initVector=coef2  distributed_mem=Distributed memory  rst=off  init_reg=0  en=off  latency=1  arith_type="Signed  (2's comp)"  n_bits=longCorr_coef_nbits  bin_pt=longCorr_coef_bp  dbl_ovrd=off  optimize=Area  use_rpm=off  block_type=sprom
      settings: xl_area=[1 1 0 1 0 0 0]  block_version=VER_STRING_GOES_HERE
  yn : Outport  OutDataType=sfix(16)  OutScaling=2^0
  xnz : Outport  Port=2  OutDataType=sfix(16)  OutScaling=2^0
  wires:
  Down Sample1/1 -> R/1
  xn/1 -> Down Sample1/1
  DAddr/1 -> R/2
  CAddr/1 -> ROM1/1
  R/1 -> xnz/1, MAC/1(a)
  MAC/1(yn) -> Down Sample2/1
  Down Sample2/1 -> yn/1
  ROM1/1 -> MAC/2(coeff)
  LD/1 -> MAC/3(ld)

== system ofdm_txrx_supermimo/OFDM Rx MIMO/Long Corr HP/C1/C16 ==
  xn : Inport  OutDataType=sfix(16)  OutScaling=2^0
  DAddr : Inport  Port=2  OutDataType=sfix(16)  OutScaling=2^0
  CAddr : Inport  Port=3  OutDataType=sfix(16)  OutScaling=2^0
  LD : Inport  Port=4  OutDataType=sfix(16)  OutScaling=2^0
  Down Sample1 : xbsIndex_r4/Down Sample  sample_ratio=length(coef2)  sample_phase=First Value of Frame  en=off  latency=0  dbl_ovrd=off  block_type=dsamp
      settings: xl_area=[3 3 0 2 0 0 0]  block_version=VER_STRING_GOES_HERE
  Down Sample2 : xbsIndex_r4/Down Sample  sample_ratio=length(coef2)  sample_phase=First Value of Frame  en=off  latency=0  dbl_ovrd=off  block_type=dsamp
      settings: xl_area=[5 5 0 4 0 0 0]  block_version=VER_STRING_GOES_HERE
  MAC : Subsystem
  R : xbsIndex_r4/Addressable Shift Register  infer_latency=off  depth=length(coef2)  initVector=[0]  en=off  dbl_ovrd=off  use_behavioral_HDL=off  use_rpm=off  block_type=addrsr
      settings: infoedit="Delay of  configurable length.  Any element  in the delay     line   can    be   addressed   and   driven    onto   the   output     port.<P><P>Hardware  notes: Implemented  using SRL16s.     If Virtex-4, Virtex-II or Spartan-3 devices are used, multiple SRLC16s are cascaded together."  xl_area=[1 0 0 2 0 0 0]  block_version=VER_STRING_GOES_HERE
  ROM1 : xbsIndex_r4/ROM  depth=length(coef2)  initVector=coef2  distributed_mem=Distributed memory  rst=off  init_reg=0  en=off  latency=1  arith_type="Signed  (2's comp)"  n_bits=longCorr_coef_nbits  bin_pt=longCorr_coef_bp  dbl_ovrd=off  optimize=Area  use_rpm=off  block_type=sprom
      settings: xl_area=[1 1 0 1 0 0 0]  block_version=VER_STRING_GOES_HERE
  yn : Outport  OutDataType=sfix(16)  OutScaling=2^0
  xnz : Outport  Port=2  OutDataType=sfix(16)  OutScaling=2^0
  wires:
  LD/1 -> MAC/3(ld)
  ROM1/1 -> MAC/2(coeff)
  Down Sample2/1 -> yn/1
  MAC/1(yn) -> Down Sample2/1
  R/1 -> xnz/1, MAC/1(a)
  CAddr/1 -> ROM1/1
  DAddr/1 -> R/2
  xn/1 -> Down Sample1/1
  Down Sample1/1 -> R/1

== system ofdm_txrx_supermimo/OFDM Rx MIMO/Long Corr HP/C1/C2 ==
  xn : Inport  OutDataType=sfix(16)  OutScaling=2^0
  DAddr : Inport  Port=2  OutDataType=sfix(16)  OutScaling=2^0
  CAddr : Inport  Port=3  OutDataType=sfix(16)  OutScaling=2^0
  LD : Inport  Port=4  OutDataType=sfix(16)  OutScaling=2^0
  Down Sample1 : xbsIndex_r4/Down Sample  sample_ratio=length(coef2)  sample_phase=First Value of Frame  en=off  latency=0  dbl_ovrd=off  block_type=dsamp
      settings: xl_area=[3 3 0 2 0 0 0]  block_version=VER_STRING_GOES_HERE
  Down Sample2 : xbsIndex_r4/Down Sample  sample_ratio=length(coef2)  sample_phase=First Value of Frame  en=off  latency=0  dbl_ovrd=off  block_type=dsamp
      settings: xl_area=[5 5 0 4 0 0 0]  block_version=VER_STRING_GOES_HERE
  MAC : Subsystem
  R : xbsIndex_r4/Addressable Shift Register  infer_latency=off  depth=length(coef2)  initVector=[0]  en=off  dbl_ovrd=off  use_behavioral_HDL=off  use_rpm=off  block_type=addrsr
      settings: infoedit="Delay of  configurable length.  Any element  in the delay     line   can    be   addressed   and   driven    onto   the   output     port.<P><P>Hardware  notes: Implemented  using SRL16s.     If Virtex-4, Virtex-II or Spartan-3 devices are used, multiple SRLC16s are cascaded together."  xl_area=[1 0 0 2 0 0 0]  block_version=VER_STRING_GOES_HERE
  ROM1 : xbsIndex_r4/ROM  depth=length(coef2)  initVector=coef2  distributed_mem=Distributed memory  rst=off  init_reg=0  en=off  latency=1  arith_type="Signed  (2's comp)"  n_bits=longCorr_coef_nbits  bin_pt=longCorr_coef_bp  dbl_ovrd=off  optimize=Area  use_rpm=off  block_type=sprom
      settings: xl_area=[1 1 0 1 0 0 0]  block_version=VER_STRING_GOES_HERE
  yn : Outport  OutDataType=sfix(16)  OutScaling=2^0
  xnz : Outport  Port=2  OutDataType=sfix(16)  OutScaling=2^0
  wires:
  Down Sample1/1 -> R/1
  xn/1 -> Down Sample1/1
  DAddr/1 -> R/2
  CAddr/1 -> ROM1/1
  R/1 -> xnz/1, MAC/1(a)
  MAC/1(yn) -> Down Sample2/1
  Down Sample2/1 -> yn/1
  ROM1/1 -> MAC/2(coeff)
  LD/1 -> MAC/3(ld)

== system ofdm_txrx_supermimo/OFDM Rx MIMO/Long Corr HP/C1/C3 ==
  xn : Inport  OutDataType=sfix(16)  OutScaling=2^0
  DAddr : Inport  Port=2  OutDataType=sfix(16)  OutScaling=2^0
  CAddr : Inport  Port=3  OutDataType=sfix(16)  OutScaling=2^0
  LD : Inport  Port=4  OutDataType=sfix(16)  OutScaling=2^0
  Down Sample1 : xbsIndex_r4/Down Sample  sample_ratio=length(coef2)  sample_phase=First Value of Frame  en=off  latency=0  dbl_ovrd=off  block_type=dsamp
      settings: xl_area=[3 3 0 2 0 0 0]  block_version=VER_STRING_GOES_HERE
  Down Sample2 : xbsIndex_r4/Down Sample  sample_ratio=length(coef2)  sample_phase=First Value of Frame  en=off  latency=0  dbl_ovrd=off  block_type=dsamp
      settings: xl_area=[5 5 0 4 0 0 0]  block_version=VER_STRING_GOES_HERE
  MAC : Subsystem
  R : xbsIndex_r4/Addressable Shift Register  infer_latency=off  depth=length(coef2)  initVector=[0]  en=off  dbl_ovrd=off  use_behavioral_HDL=off  use_rpm=off  block_type=addrsr
      settings: infoedit="Delay of  configurable length.  Any element  in the delay     line   can    be   addressed   and   driven    onto   the   output     port.<P><P>Hardware  notes: Implemented  using SRL16s.     If Virtex-4, Virtex-II or Spartan-3 devices are used, multiple SRLC16s are cascaded together."  xl_area=[1 0 0 2 0 0 0]  block_version=VER_STRING_GOES_HERE
  ROM1 : xbsIndex_r4/ROM  depth=length(coef2)  initVector=coef2  distributed_mem=Distributed memory  rst=off  init_reg=0  en=off  latency=1  arith_type="Signed  (2's comp)"  n_bits=longCorr_coef_nbits  bin_pt=longCorr_coef_bp  dbl_ovrd=off  optimize=Area  use_rpm=off  block_type=sprom
      settings: xl_area=[1 1 0 1 0 0 0]  block_version=VER_STRING_GOES_HERE
  yn : Outport  OutDataType=sfix(16)  OutScaling=2^0
  xnz : Outport  Port=2  OutDataType=sfix(16)  OutScaling=2^0
  wires:
  Down Sample1/1 -> R/1
  xn/1 -> Down Sample1/1
  DAddr/1 -> R/2
  CAddr/1 -> ROM1/1
  R/1 -> xnz/1, MAC/1(a)
  MAC/1(yn) -> Down Sample2/1
  Down Sample2/1 -> yn/1
  ROM1/1 -> MAC/2(coeff)
  LD/1 -> MAC/3(ld)

== system ofdm_txrx_supermimo/OFDM Rx MIMO/Long Corr HP/C1/C4 ==
  xn : Inport  OutDataType=sfix(16)  OutScaling=2^0
  DAddr : Inport  Port=2  OutDataType=sfix(16)  OutScaling=2^0
  CAddr : Inport  Port=3  OutDataType=sfix(16)  OutScaling=2^0
  LD : Inport  Port=4  OutDataType=sfix(16)  OutScaling=2^0
  Down Sample1 : xbsIndex_r4/Down Sample  sample_ratio=length(coef2)  sample_phase=First Value of Frame  en=off  latency=0  dbl_ovrd=off  block_type=dsamp
      settings: xl_area=[3 3 0 2 0 0 0]  block_version=VER_STRING_GOES_HERE
  Down Sample2 : xbsIndex_r4/Down Sample  sample_ratio=length(coef2)  sample_phase=First Value of Frame  en=off  latency=0  dbl_ovrd=off  block_type=dsamp
      settings: xl_area=[5 5 0 4 0 0 0]  block_version=VER_STRING_GOES_HERE
  MAC : Subsystem
  R : xbsIndex_r4/Addressable Shift Register  infer_latency=off  depth=length(coef2)  initVector=[0]  en=off  dbl_ovrd=off  use_behavioral_HDL=off  use_rpm=off  block_type=addrsr
      settings: infoedit="Delay of  configurable length.  Any element  in the delay     line   can    be   addressed   and   driven    onto   the   output     port.<P><P>Hardware  notes: Implemented  using SRL16s.     If Virtex-4, Virtex-II or Spartan-3 devices are used, multiple SRLC16s are cascaded together."  xl_area=[1 0 0 2 0 0 0]  block_version=VER_STRING_GOES_HERE
  ROM1 : xbsIndex_r4/ROM  depth=length(coef2)  initVector=coef2  distributed_mem=Distributed memory  rst=off  init_reg=0  en=off  latency=1  arith_type="Signed  (2's comp)"  n_bits=longCorr_coef_nbits  bin_pt=longCorr_coef_bp  dbl_ovrd=off  optimize=Area  use_rpm=off  block_type=sprom
      settings: xl_area=[1 1 0 1 0 0 0]  block_version=VER_STRING_GOES_HERE
  yn : Outport  OutDataType=sfix(16)  OutScaling=2^0
  xnz : Outport  Port=2  OutDataType=sfix(16)  OutScaling=2^0
  wires:
  Down Sample1/1 -> R/1
  xn/1 -> Down Sample1/1
  DAddr/1 -> R/2
  CAddr/1 -> ROM1/1
  R/1 -> xnz/1, MAC/1(a)
  MAC/1(yn) -> Down Sample2/1
  Down Sample2/1 -> yn/1
  ROM1/1 -> MAC/2(coeff)
  LD/1 -> MAC/3(ld)

== system ofdm_txrx_supermimo/OFDM Rx MIMO/Long Corr HP/C1/C5 ==
  xn : Inport  OutDataType=sfix(16)  OutScaling=2^0
  DAddr : Inport  Port=2  OutDataType=sfix(16)  OutScaling=2^0
  CAddr : Inport  Port=3  OutDataType=sfix(16)  OutScaling=2^0
  LD : Inport  Port=4  OutDataType=sfix(16)  OutScaling=2^0
  Down Sample1 : xbsIndex_r4/Down Sample  sample_ratio=length(coef2)  sample_phase=First Value of Frame  en=off  latency=0  dbl_ovrd=off  block_type=dsamp
      settings: xl_area=[3 3 0 2 0 0 0]  block_version=VER_STRING_GOES_HERE
  Down Sample2 : xbsIndex_r4/Down Sample  sample_ratio=length(coef2)  sample_phase=First Value of Frame  en=off  latency=0  dbl_ovrd=off  block_type=dsamp
      settings: xl_area=[5 5 0 4 0 0 0]  block_version=VER_STRING_GOES_HERE
  MAC : Subsystem
  R : xbsIndex_r4/Addressable Shift Register  infer_latency=off  depth=length(coef2)  initVector=[0]  en=off  dbl_ovrd=off  use_behavioral_HDL=off  use_rpm=off  block_type=addrsr
      settings: infoedit="Delay of  configurable length.  Any element  in the delay     line   can    be   addressed   and   driven    onto   the   output     port.<P><P>Hardware  notes: Implemented  using SRL16s.     If Virtex-4, Virtex-II or Spartan-3 devices are used, multiple SRLC16s are cascaded together."  xl_area=[1 0 0 2 0 0 0]  block_version=VER_STRING_GOES_HERE
  ROM1 : xbsIndex_r4/ROM  depth=length(coef2)  initVector=coef2  distributed_mem=Distributed memory  rst=off  init_reg=0  en=off  latency=1  arith_type="Signed  (2's comp)"  n_bits=longCorr_coef_nbits  bin_pt=longCorr_coef_bp  dbl_ovrd=off  optimize=Area  use_rpm=off  block_type=sprom
      settings: xl_area=[1 1 0 1 0 0 0]  block_version=VER_STRING_GOES_HERE
  yn : Outport  OutDataType=sfix(16)  OutScaling=2^0
  xnz : Outport  Port=2  OutDataType=sfix(16)  OutScaling=2^0
  wires:
  Down Sample1/1 -> R/1
  xn/1 -> Down Sample1/1
  DAddr/1 -> R/2
  CAddr/1 -> ROM1/1
  R/1 -> xnz/1, MAC/1(a)
  MAC/1(yn) -> Down Sample2/1
  Down Sample2/1 -> yn/1
  ROM1/1 -> MAC/2(coeff)
  LD/1 -> MAC/3(ld)

== system ofdm_txrx_supermimo/OFDM Rx MIMO/Long Corr HP/C1/C6 ==
  xn : Inport  OutDataType=sfix(16)  OutScaling=2^0
  DAddr : Inport  Port=2  OutDataType=sfix(16)  OutScaling=2^0
  CAddr : Inport  Port=3  OutDataType=sfix(16)  OutScaling=2^0
  LD : Inport  Port=4  OutDataType=sfix(16)  OutScaling=2^0
  Down Sample1 : xbsIndex_r4/Down Sample  sample_ratio=length(coef2)  sample_phase=First Value of Frame  en=off  latency=0  dbl_ovrd=off  block_type=dsamp
      settings: xl_area=[3 3 0 2 0 0 0]  block_version=VER_STRING_GOES_HERE
  Down Sample2 : xbsIndex_r4/Down Sample  sample_ratio=length(coef2)  sample_phase=First Value of Frame  en=off  latency=0  dbl_ovrd=off  block_type=dsamp
      settings: xl_area=[5 5 0 4 0 0 0]  block_version=VER_STRING_GOES_HERE
  MAC : Subsystem
  R : xbsIndex_r4/Addressable Shift Register  infer_latency=off  depth=length(coef2)  initVector=[0]  en=off  dbl_ovrd=off  use_behavioral_HDL=off  use_rpm=off  block_type=addrsr
      settings: infoedit="Delay of  configurable length.  Any element  in the delay     line   can    be   addressed   and   driven    onto   the   output     port.<P><P>Hardware  notes: Implemented  using SRL16s.     If Virtex-4, Virtex-II or Spartan-3 devices are used, multiple SRLC16s are cascaded together."  xl_area=[1 0 0 2 0 0 0]  block_version=VER_STRING_GOES_HERE
  ROM1 : xbsIndex_r4/ROM  depth=length(coef2)  initVector=coef2  distributed_mem=Distributed memory  rst=off  init_reg=0  en=off  latency=1  arith_type="Signed  (2's comp)"  n_bits=longCorr_coef_nbits  bin_pt=longCorr_coef_bp  dbl_ovrd=off  optimize=Area  use_rpm=off  block_type=sprom
      settings: xl_area=[1 1 0 1 0 0 0]  block_version=VER_STRING_GOES_HERE
  yn : Outport  OutDataType=sfix(16)  OutScaling=2^0
  xnz : Outport  Port=2  OutDataType=sfix(16)  OutScaling=2^0
  wires:
  Down Sample1/1 -> R/1
  xn/1 -> Down Sample1/1
  DAddr/1 -> R/2
  CAddr/1 -> ROM1/1
  R/1 -> xnz/1, MAC/1(a)
  MAC/1(yn) -> Down Sample2/1
  Down Sample2/1 -> yn/1
  ROM1/1 -> MAC/2(coeff)
  LD/1 -> MAC/3(ld)

== system ofdm_txrx_supermimo/OFDM Rx MIMO/Long Corr HP/C1/C7 ==
  xn : Inport  OutDataType=sfix(16)  OutScaling=2^0
  DAddr : Inport  Port=2  OutDataType=sfix(16)  OutScaling=2^0
  CAddr : Inport  Port=3  OutDataType=sfix(16)  OutScaling=2^0
  LD : Inport  Port=4  OutDataType=sfix(16)  OutScaling=2^0
  Down Sample1 : xbsIndex_r4/Down Sample  sample_ratio=length(coef2)  sample_phase=First Value of Frame  en=off  latency=0  dbl_ovrd=off  block_type=dsamp
      settings: xl_area=[3 3 0 2 0 0 0]  block_version=VER_STRING_GOES_HERE
  Down Sample2 : xbsIndex_r4/Down Sample  sample_ratio=length(coef2)  sample_phase=First Value of Frame  en=off  latency=0  dbl_ovrd=off  block_type=dsamp
      settings: xl_area=[5 5 0 4 0 0 0]  block_version=VER_STRING_GOES_HERE
  MAC : Subsystem
  R : xbsIndex_r4/Addressable Shift Register  infer_latency=off  depth=length(coef2)  initVector=[0]  en=off  dbl_ovrd=off  use_behavioral_HDL=off  use_rpm=off  block_type=addrsr
      settings: infoedit="Delay of  configurable length.  Any element  in the delay     line   can    be   addressed   and   driven    onto   the   output     port.<P><P>Hardware  notes: Implemented  using SRL16s.     If Virtex-4, Virtex-II or Spartan-3 devices are used, multiple SRLC16s are cascaded together."  xl_area=[1 0 0 2 0 0 0]  block_version=VER_STRING_GOES_HERE
  ROM1 : xbsIndex_r4/ROM  depth=length(coef2)  initVector=coef2  distributed_mem=Distributed memory  rst=off  init_reg=0  en=off  latency=1  arith_type="Signed  (2's comp)"  n_bits=longCorr_coef_nbits  bin_pt=longCorr_coef_bp  dbl_ovrd=off  optimize=Area  use_rpm=off  block_type=sprom
      settings: xl_area=[1 1 0 1 0 0 0]  block_version=VER_STRING_GOES_HERE
  yn : Outport  OutDataType=sfix(16)  OutScaling=2^0
  xnz : Outport  Port=2  OutDataType=sfix(16)  OutScaling=2^0
  wires:
  Down Sample1/1 -> R/1
  xn/1 -> Down Sample1/1
  DAddr/1 -> R/2
  CAddr/1 -> ROM1/1
  R/1 -> xnz/1, MAC/1(a)
  MAC/1(yn) -> Down Sample2/1
  Down Sample2/1 -> yn/1
  ROM1/1 -> MAC/2(coeff)
  LD/1 -> MAC/3(ld)
